simulink model "Simulatore_COMAU_UBHAND_IV_manipolazione_interna"  (Simulink 7.6)
settings: Solver=ode45  StartTime=0.0  StopTime=inf  RelTol=1e-3
values from neighbouring files (symbols this model uses):
  a_f = zeros(4,5)    [KinematicData.m]
  alpha_f = zeros(4,5)    [KinematicData.m]
  d_f = zeros(4,5)    [KinematicData.m]
  dc4_1 = 660    [KinematicData.m]
  T6HAND = [1 0 0 0;0 1 0 0; 0 0 1 357.48+95; 0 0 0 1]    [KinematicData.m]
  teta_d = atan(647.07/130)    [KinematicData.m]
  teta_f = zeros(4,5)    [KinematicData.m]
undefined symbols (not defined in the file or in its folder): a_c, a_w, alpha_c, alpha_w, d_c, d_w, Dt_fingers, teta_c
libraries: simulink x281, dspmtrx3 x171, aerolibutil x163, aerolibconvert2 x64, simulink_extras x46, vrlib x26, aerolibtransform2 x7, dspnetwork x1
(part 10 of 15)

== system Simulatore_COMAU_UBHAND_IV_manipolazione_interna/Work Space Control/Grasp Planner/box/punti di contatto box/punti di contatto sfera/Subsystem3 ==
  dimens1/2/side : Inport
  T_object : Inport  Port=2
  Constant : Constant
  Demux : Demux  Outputs=3
  Demux2 : Demux  Outputs=3
  Demux3 : Demux  Outputs=3
  Display : Display
  Display1 : Display
  Gain : Gain  Gain=-1
  Gain1 : Gain  Gain=-1
  Gain2 : Gain  Gain=-1
  Gain3 : Gain  Gain=1/2
  Matrix Multiply : Product  Multiplication=Matrix(*)
  Multiport Switch : MultiPortSwitch  Inputs=6  zeroidx=off
  Mux : Mux  Inputs=2
  Mux{1..6} (6 alike) : Mux  Inputs=3
  R_0EE1 : dspmtrx3/Submatrix  RowSpan=Range of rows  RowStartMode=Index  RowStartIndex=1  RowEndMode=Index  RowEndIndex=3  ColSpan=One column  ColStartMode=Index  ColStartIndex=4  ColEndMode=Index  ColEndIndex=4
  R_0EE2 : dspmtrx3/Submatrix  RowSpan=Range of rows  RowStartMode=Index  RowStartIndex=1  RowEndMode=Index  RowEndIndex=3  ColSpan=Range of columns  ColStartMode=Index  ColStartIndex=1  ColEndMode=Index  ColEndIndex=3
  Sum : Sum  Inputs=|++
  Sum3 : Sum
  Sum4 : Sum  Inputs=|++
  Sum5 : Sum  Inputs=|++
  dimens1/2/side1 : InportShadow  Port=1
  xyz 0 : Outport
  wires:
  R_0EE1/1 -> Sum3/2
  R_0EE2/1 -> Matrix Multiply/1
  T_object/1 -> R_0EE1/1, R_0EE2/1
  Multiport Switch/1 -> Matrix Multiply/2
  Matrix Multiply/1 -> Sum3/1
  [dim2] -> Mux5/2
  Gain1/1 -> Mux6/3
  [dim2] -> Mux6/2
  Gain3/1 -> Display1/1, Demux/1
  Demux/1 -> Sum5/1
  Demux/3 -> Sum/2
  [dim2] -> Mux3/3
  [dim2] -> Mux4/3
  Gain2/1 -> Mux4/2
  Mux4/1 -> Multiport Switch/7
  Mux3/1 -> Multiport Switch/6
  Mux6/1 -> Multiport Switch/5
  Mux5/1 -> Multiport Switch/4
  Mux2/1 -> Multiport Switch/3
  Mux1/1 -> Multiport Switch/2
  [dim1] -> Mux4/1
  [dim1] -> Mux3/1
  [dim1] -> Mux6/1
  [dim1] -> Mux5/1
  [dim1] -> Mux2/2
  [dim2] -> Mux2/3
  [dim1] -> Mux1/2
  [dim2] -> Mux1/3
  Demux3/1 -> [dim1]
  Demux3/2 -> [dim2]
  [radius_spheres] -> Sum5/2
  Sum5/1 -> Mux3/2, Gain2/1
  Sum4/1 -> Mux5/3, Gain1/1
  Demux/2 -> Sum4/2
  [radius_spheres] -> Sum4/1
  Gain/1 -> Mux2/1
  Sum/1 -> Display/1, Gain/1, Mux1/1
  dimens1/2/side1/1 -> Demux3/1
  Demux2/3 -> Multiport Switch/1
  Constant/1 -> Mux/2
  dimens1/2/side/1 -> Demux2/1
  Sum3/1 -> Mux/1
  Mux/1 -> xyz 0/1
  [radius_spheres] -> Sum/1
  [box_size] -> Gain3/1

== system Simulatore_COMAU_UBHAND_IV_manipolazione_interna/Work Space Control/Grasp Planner/box/punti di contatto box/punti di contatto sfera/Subsystem4 ==
  dimens1/2/side : Inport
  T_object : Inport  Port=2
  Constant : Constant
  Demux : Demux  Outputs=3
  Demux2 : Demux  Outputs=3
  Demux3 : Demux  Outputs=3
  Display : Display
  Display1 : Display
  Gain : Gain  Gain=-1
  Gain1 : Gain  Gain=-1
  Gain2 : Gain  Gain=-1
  Gain3 : Gain  Gain=1/2
  Matrix Multiply : Product  Multiplication=Matrix(*)
  Multiport Switch : MultiPortSwitch  Inputs=6  zeroidx=off
  Mux : Mux  Inputs=2
  Mux{1..6} (6 alike) : Mux  Inputs=3
  R_0EE1 : dspmtrx3/Submatrix  RowSpan=Range of rows  RowStartMode=Index  RowStartIndex=1  RowEndMode=Index  RowEndIndex=3  ColSpan=One column  ColStartMode=Index  ColStartIndex=4  ColEndMode=Index  ColEndIndex=4
  R_0EE2 : dspmtrx3/Submatrix  RowSpan=Range of rows  RowStartMode=Index  RowStartIndex=1  RowEndMode=Index  RowEndIndex=3  ColSpan=Range of columns  ColStartMode=Index  ColStartIndex=1  ColEndMode=Index  ColEndIndex=3
  Sum : Sum  Inputs=|++
  Sum3 : Sum
  Sum4 : Sum  Inputs=|++
  Sum5 : Sum  Inputs=|++
  dimens1/2/side1 : InportShadow  Port=1
  xyz 0 : Outport
  wires:
  [box_size] -> Gain3/1
  [radius_spheres] -> Sum/1
  Mux/1 -> xyz 0/1
  Sum3/1 -> Mux/1
  dimens1/2/side/1 -> Demux2/1
  Constant/1 -> Mux/2
  Demux2/3 -> Multiport Switch/1
  dimens1/2/side1/1 -> Demux3/1
  Sum/1 -> Mux1/1, Gain/1, Display/1
  Gain/1 -> Mux2/1
  [radius_spheres] -> Sum4/1
  Demux/2 -> Sum4/2
  Sum4/1 -> Gain1/1, Mux5/3
  Sum5/1 -> Gain2/1, Mux3/2
  [radius_spheres] -> Sum5/2
  Demux3/2 -> [dim2]
  Demux3/1 -> [dim1]
  [dim2] -> Mux1/3
  [dim1] -> Mux1/2
  [dim2] -> Mux2/3
  [dim1] -> Mux2/2
  [dim1] -> Mux5/1
  [dim1] -> Mux6/1
  [dim1] -> Mux3/1
  [dim1] -> Mux4/1
  Mux1/1 -> Multiport Switch/2
  Mux2/1 -> Multiport Switch/3
  Mux5/1 -> Multiport Switch/4
  Mux6/1 -> Multiport Switch/5
  Mux3/1 -> Multiport Switch/6
  Mux4/1 -> Multiport Switch/7
  Gain2/1 -> Mux4/2
  [dim2] -> Mux4/3
  [dim2] -> Mux3/3
  Demux/3 -> Sum/2
  Demux/1 -> Sum5/1
  Gain3/1 -> Demux/1, Display1/1
  [dim2] -> Mux6/2
  Gain1/1 -> Mux6/3
  [dim2] -> Mux5/2
  Matrix Multiply/1 -> Sum3/1
  Multiport Switch/1 -> Matrix Multiply/2
  T_object/1 -> R_0EE2/1, R_0EE1/1
  R_0EE2/1 -> Matrix Multiply/1
  R_0EE1/1 -> Sum3/2

== system Simulatore_COMAU_UBHAND_IV_manipolazione_interna/Work Space Control/Grasp Planner/cilindro/Subsystem/R_end_effector/rot_x ==
  alpha : Inport
  Angle Conversion : aerolibconvert2/Angle Conversion  IU=deg  OU=rad
  Constant{,1..2,5} (4 alike) : Constant  Value=0
  Constant4 : Constant
  Create 3x3 Matrix : aerolibutil/Create 3x3 Matrix
  Gain : Gain  Gain=-1
  Trigonometric Function : Trigonometry
  Trigonometric Function1 : Trigonometry  Operator=cos
  Trigonometric Function2 : Trigonometry
  Trigonometric Function3 : Trigonometry  Operator=cos
  rotx : Outport
  wires:
  alpha/1 -> Angle Conversion/1
  Angle Conversion/1 -> Trigonometric Function3/1, Trigonometric Function2/1, Trigonometric Function/1, Trigonometric Function1/1
  Create 3x3 Matrix/1 -> rotx/1
  Constant4/1 -> Create 3x3 Matrix/1
  Constant2/1 -> Create 3x3 Matrix/2
  Constant/1 -> Create 3x3 Matrix/3
  Constant5/1 -> Create 3x3 Matrix/4
  Trigonometric Function3/1 -> Create 3x3 Matrix/5
  Trigonometric Function2/1 -> Gain/1
  Gain/1 -> Create 3x3 Matrix/6
  Constant1/1 -> Create 3x3 Matrix/7
  Trigonometric Function/1 -> Create 3x3 Matrix/8
  Trigonometric Function1/1 -> Create 3x3 Matrix/9

== system Simulatore_COMAU_UBHAND_IV_manipolazione_interna/Work Space Control/Grasp Planner/cilindro/Subsystem/R_end_effector/rot_y ==
  beta : Inport
  Angle Conversion : aerolibconvert2/Angle Conversion  IU=deg  OU=rad
  Constant{1..3,5} (4 alike) : Constant  Value=0
  Constant4 : Constant
  Create 3x3 Matrix : aerolibutil/Create 3x3 Matrix
  Gain : Gain  Gain=-1
  Trigonometric Function : Trigonometry
  Trigonometric Function1 : Trigonometry  Operator=cos
  Trigonometric Function4 : Trigonometry  Operator=cos
  Trigonometric Function5 : Trigonometry
  roty : Outport
  wires:
  Constant1/1 -> Create 3x3 Matrix/8
  Gain/1 -> Create 3x3 Matrix/7
  Trigonometric Function4/1 -> Create 3x3 Matrix/1
  Trigonometric Function1/1 -> Create 3x3 Matrix/9
  Trigonometric Function/1 -> Gain/1
  Constant3/1 -> Create 3x3 Matrix/6
  Constant4/1 -> Create 3x3 Matrix/5
  Constant5/1 -> Create 3x3 Matrix/4
  Trigonometric Function5/1 -> Create 3x3 Matrix/3
  Constant2/1 -> Create 3x3 Matrix/2
  Create 3x3 Matrix/1 -> roty/1
  Angle Conversion/1 -> Trigonometric Function4/1, Trigonometric Function5/1, Trigonometric Function/1, Trigonometric Function1/1
  beta/1 -> Angle Conversion/1

== system Simulatore_COMAU_UBHAND_IV_manipolazione_interna/Work Space Control/Grasp Planner/cilindro/Subsystem/R_end_effector/rot_z ==
  gamma : Inport
  Angle Conversion : aerolibconvert2/Angle Conversion  IU=deg  OU=rad
  Constant{,1..3} (4 alike) : Constant  Value=0
  Constant4 : Constant
  Create 3x3 Matrix : aerolibutil/Create 3x3 Matrix
  Gain1 : Gain  Gain=-1
  Trigonometric Function3 : Trigonometry  Operator=cos
  Trigonometric Function4 : Trigonometry  Operator=cos
  Trigonometric Function5 : Trigonometry
  Trigonometric Function6 : Trigonometry
  rotz : Outport
  wires:
  Trigonometric Function5/1 -> Gain1/1
  Constant4/1 -> Create 3x3 Matrix/9
  Constant3/1 -> Create 3x3 Matrix/8
  Constant1/1 -> Create 3x3 Matrix/7
  Constant2/1 -> Create 3x3 Matrix/6
  Trigonometric Function3/1 -> Create 3x3 Matrix/5
  Trigonometric Function6/1 -> Create 3x3 Matrix/4
  Constant/1 -> Create 3x3 Matrix/3
  Gain1/1 -> Create 3x3 Matrix/2
  Trigonometric Function4/1 -> Create 3x3 Matrix/1
  Create 3x3 Matrix/1 -> rotz/1
  Angle Conversion/1 -> Trigonometric Function4/1, Trigonometric Function5/1, Trigonometric Function6/1, Trigonometric Function3/1
  gamma/1 -> Angle Conversion/1

== system Simulatore_COMAU_UBHAND_IV_manipolazione_interna/Work Space Control/Grasp Planner/cilindro/punti di contatto cilindro/object rotation matrix/R ==
  alpha : Inport
  beta : Inport  Port=2
  gamma : Inport  Port=3
  Matrix Multiply3 : Product  Multiplication=Matrix(*)
  Matrix Multiply4 : Product  Multiplication=Matrix(*)
  rot_x : Subsystem
  rot_y : Subsystem
  rot_z : Subsystem
  R : Outport
  wires:
  gamma/1 -> rot_z/1(gamma)
  beta/1 -> rot_y/1(beta)
  alpha/1 -> rot_x/1(alpha)
  rot_x/1(rotx) -> Matrix Multiply3/1
  rot_y/1(roty) -> Matrix Multiply3/2
  rot_z/1(rotz) -> Matrix Multiply4/2
  Matrix Multiply3/1 -> Matrix Multiply4/1
  Matrix Multiply4/1 -> R/1

== system Simulatore_COMAU_UBHAND_IV_manipolazione_interna/Work Space Control/Grasp Planner/cilindro/punti di contatto cilindro/object rotation matrix/R_01 ==
  alpha : Inport
  beta : Inport  Port=2
  gamma : Inport  Port=3
  Matrix Multiply3 : Product  Multiplication=Matrix(*)
  Matrix Multiply4 : Product  Multiplication=Matrix(*)
  rot_x : Subsystem
  rot_y : Subsystem
  rot_z : Subsystem
  R_01 : Outport
  wires:
  gamma/1 -> rot_z/1(gamma)
  beta/1 -> rot_y/1(beta)
  alpha/1 -> rot_x/1(alpha)
  rot_x/1(rotx) -> Matrix Multiply3/1
  rot_y/1(roty) -> Matrix Multiply3/2
  rot_z/1(rotz) -> Matrix Multiply4/2
  Matrix Multiply3/1 -> Matrix Multiply4/1
  Matrix Multiply4/1 -> R_01/1

== system Simulatore_COMAU_UBHAND_IV_manipolazione_interna/Work Space Control/Grasp Planner/cilindro/punti di contatto cilindro/object rotation matrix/R_56 ==
  alpha : Inport
  beta : Inport  Port=2
  gamma : Inport  Port=3
  Matrix Multiply3 : Product  Multiplication=Matrix(*)
  Matrix Multiply4 : Product  Multiplication=Matrix(*)
  rot_x : Subsystem
  rot_y : Subsystem
  rot_z : Subsystem
  R56 : Outport
  wires:
  Matrix Multiply4/1 -> R56/1
  Matrix Multiply3/1 -> Matrix Multiply4/1
  rot_z/1(rotz) -> Matrix Multiply4/2
  rot_y/1(roty) -> Matrix Multiply3/2
  rot_x/1(rotx) -> Matrix Multiply3/1
  alpha/1 -> rot_x/1(alpha)
  beta/1 -> rot_y/1(beta)
  gamma/1 -> rot_z/1(gamma)

== system Simulatore_COMAU_UBHAND_IV_manipolazione_interna/Work Space Control/Grasp Planner/cilindro/punti di contatto cilindro/object rotation matrix/Subsystem ==
  R : Inport
  p : Inport  Port=2
  Constant1 : Constant  Value=[0 0 0 1]  VectorParams1D=off
  Matrix Concatenate : Concatenate  Mode=Multidimensional array  ConcatenateDimension=2
  Matrix Concatenate1 : Concatenate  Mode=Multidimensional array
  T : Outport
  wires:
  Constant1/1 -> Matrix Concatenate1/2
  Matrix Concatenate/1 -> Matrix Concatenate1/1
  p/1 -> Matrix Concatenate/2
  R/1 -> Matrix Concatenate/1
  Matrix Concatenate1/1 -> T/1

== system Simulatore_COMAU_UBHAND_IV_manipolazione_interna/Work Space Control/Grasp Planner/cilindro/punti di contatto cilindro/object rotation matrix/Subsystem1 ==
  R : Inport
  p : Inport  Port=2
  Constant2 : Constant  Value=[0 0 0 1]  VectorParams1D=off
  Matrix Concatenate2 : Concatenate  Mode=Multidimensional array  ConcatenateDimension=2
  Matrix Concatenate3 : Concatenate  Mode=Multidimensional array
  T : Outport
  wires:
  Constant2/1 -> Matrix Concatenate3/2
  Matrix Concatenate2/1 -> Matrix Concatenate3/1
  R/1 -> Matrix Concatenate2/1
  p/1 -> Matrix Concatenate2/2
  Matrix Concatenate3/1 -> T/1

== system Simulatore_COMAU_UBHAND_IV_manipolazione_interna/Work Space Control/Grasp Planner/cilindro/punti di contatto cilindro/object rotation matrix/Subsystem2 ==
  R : Inport
  p : Inport  Port=2
  Constant1 : Constant  Value=[0 0 0 1]  VectorParams1D=off
  Matrix Concatenate : Concatenate  Mode=Multidimensional array  ConcatenateDimension=2
  Matrix Concatenate1 : Concatenate  Mode=Multidimensional array
  T : Outport
  wires:
  Matrix Concatenate1/1 -> T/1
  R/1 -> Matrix Concatenate/1
  p/1 -> Matrix Concatenate/2
  Matrix Concatenate/1 -> Matrix Concatenate1/1
  Constant1/1 -> Matrix Concatenate1/2

== system Simulatore_COMAU_UBHAND_IV_manipolazione_interna/Work Space Control/Grasp Planner/cilindro/punti di contatto cilindro/object rotation matrix/definizione_T_0_object_tip1 ==
  cilindro_pos : Inport
  R_01 : Inport  Port=2
  1pc : Inport  Port=3
  2pr : Inport  Port=4
  3pc : Inport  Port=5
  R : Inport  Port=6
  1pc1 : InportShadow  Port=3
  Display : Display
  Display1 : Display
  Matrix Multiply{1..6,8..9} (8 alike) : Product  Multiplication=Matrix(*)
  Multiport Switch1 : MultiPortSwitch  Inputs=2  zeroidx=off
  R_1 : Constant  Value=eye(3)
  R_12 : Constant  Value=eye(3)
  Sum1 : Sum
  Sum3 : Sum  Inputs=+-
  Sum4 : Sum
  Sum5 : Sum
  0pc : Outport
  0pr : Outport  Port=2
  0pc' : Outport  Port=3
  R1 : Outport  Port=4
  R_05 : Outport  Port=5
  tag scope: reference_point_cilindro=global
  wires:
  R_01/1 -> Matrix Multiply9/2, Matrix Multiply1/1, Matrix Multiply2/1, Matrix Multiply3/1, Multiport Switch1/2
  1pc/1 -> Matrix Multiply1/2
  cilindro_pos/1 -> Sum3/1, Display/1
  Matrix Multiply1/1 -> Sum3/2, Display1/1
  Sum3/1 -> 0pc/1, Sum4/1
  R_12/1 -> Matrix Multiply2/2
  Matrix Multiply2/1 -> Matrix Multiply5/1
  2pr/1 -> Matrix Multiply3/2
  Matrix Multiply3/1 -> Sum4/2
  Sum4/1 -> 0pr/1, Sum5/1
  R_1/1 -> Matrix Multiply5/2
  Sum5/1 -> 0pc'/1, Sum1/1
  Matrix Multiply8/1 -> Sum5/2
  Matrix Multiply9/1 -> R_05/1, Multiport Switch1/3
  R/1 -> Matrix Multiply8/1, Matrix Multiply9/1, R1/1
  3pc/1 -> Matrix Multiply6/2
  Matrix Multiply6/1 -> Matrix Multiply8/2
  Sum1/1 -> [reference_point_cilindro]
  Matrix Multiply4/1 -> Sum1/2
  1pc1/1 -> Matrix Multiply4/2
  Matrix Multiply5/1 -> Matrix Multiply6/1
  [orientation_mode_cilindro] -> Multiport Switch1/1
  Multiport Switch1/1 -> Matrix Multiply4/1

== system Simulatore_COMAU_UBHAND_IV_manipolazione_interna/Work Space Control/Grasp Planner/cilindro/punti di contatto cilindro/object rotation matrix/definizione_T_0_object_tip2 ==
  cilindro_pos : Inport
  R_01 : Inport  Port=2
  1pc : Inport  Port=3
  2pr : Inport  Port=4
  3pc : Inport  Port=5
  R : Inport  Port=6
  1pc1 : InportShadow  Port=3
  Matrix Multiply{1..6,8..9} (8 alike) : Product  Multiplication=Matrix(*)
  Multiport Switch1 : MultiPortSwitch  Inputs=2  zeroidx=off
  R_1 : Constant  Value=eye(3)
  R_12 : Constant  Value=eye(3)
  Sum1 : Sum
  Sum3 : Sum  Inputs=+-
  Sum4 : Sum
  Sum5 : Sum
  0pc : Outport
  0pr : Outport  Port=2
  0pc' : Outport  Port=3
  R1 : Outport  Port=4
  R_05 : Outport  Port=5
  wires:
  Multiport Switch1/1 -> Matrix Multiply4/1
  [orientation_mode_cilindro] -> Multiport Switch1/1
  Matrix Multiply5/1 -> Matrix Multiply6/1
  1pc1/1 -> Matrix Multiply4/2
  Matrix Multiply4/1 -> Sum1/2
  Matrix Multiply6/1 -> Matrix Multiply8/2
  3pc/1 -> Matrix Multiply6/2
  R/1 -> R1/1, Matrix Multiply9/1, Matrix Multiply8/1
  Matrix Multiply9/1 -> Multiport Switch1/3, R_05/1
  Matrix Multiply8/1 -> Sum5/2
  Sum5/1 -> Sum1/1, 0pc'/1
  R_1/1 -> Matrix Multiply5/2
  Sum4/1 -> Sum5/1, 0pr/1
  Matrix Multiply3/1 -> Sum4/2
  2pr/1 -> Matrix Multiply3/2
  Matrix Multiply2/1 -> Matrix Multiply5/1
  R_12/1 -> Matrix Multiply2/2
  Sum3/1 -> Sum4/1, 0pc/1
  Matrix Multiply1/1 -> Sum3/2
  cilindro_pos/1 -> Sum3/1
  1pc/1 -> Matrix Multiply1/2
  R_01/1 -> Multiport Switch1/2, Matrix Multiply3/1, Matrix Multiply2/1, Matrix Multiply1/1, Matrix Multiply9/2

== system Simulatore_COMAU_UBHAND_IV_manipolazione_interna/Work Space Control/Grasp Planner/cilindro/punti di contatto cilindro/punti di contatto cilindro/Subsystem1 ==
  teta/z : Inport
  T_object : Inport  Port=2
  offset : Inport  Port=3
  object_manipulation : Inport  Port=4
  Constant : Constant
  Constant1 : Constant
  Constant2 : Constant  Value=eye(3,3)  VectorParams1D=off
  Constant4 : Constant
  Demux : Demux  Outputs=2
  Demux2 : Demux  Outputs=2
  Display{,1..3} (4 alike) : Display
  Gain : Gain  Gain=1/2
  Matrix Multiply{1..5} (5 alike) : Product  Multiplication=Matrix(*)
  Mux1 : Mux  Inputs=2
  Mux2 : Mux  Inputs=2
  Mux3 : Mux  Inputs=3
  Polar to Cartesian : simulink_extras/Transformations/Polar to Cartesian
  Subsystem1 : Subsystem
  Sum : Sum  Inputs=|+++
  T^-1 : Subsystem
  index_xyz_0 : Outport
  xyz 0 : Outport  Port=2
  wires:
  T_object/1 -> Matrix Multiply1/1, Matrix Multiply4/1
  Polar to Cartesian/1 -> Mux3/1
  Polar to Cartesian/2 -> Mux3/2
  Demux2/2 -> Mux3/3
  teta/z/1 -> Demux2/1
  Demux2/1 -> Polar to Cartesian/2
  [radius_spheres] -> Gain/1
  Demux/2 -> Sum/2
  Sum/1 -> Polar to Cartesian/1
  [cilindro_size] -> Demux/1
  Constant1/1 -> Mux2/2
  Gain/1 -> Sum/1
  offset/1 -> Sum/3
  Constant/1 -> [one]
  Mux3/1 -> Mux1/1, Mux2/1
  Matrix Multiply3/1 -> Display1/1, xyz 0/1
  Constant2/1 -> Subsystem1/1(R)
  [reference_point] -> Subsystem1/2(p)
  Subsystem1/1(T) -> T^-1/1(T), Matrix Multiply4/2
  T^-1/1(T^-1) -> Matrix Multiply2/1
  object_manipulation/1 -> Matrix Multiply5/1
  Matrix Multiply2/1 -> Matrix Multiply5/2, Display2/1
  Constant4/1 -> Mux1/2
  Mux1/1 -> Matrix Multiply2/2
  Matrix Multiply5/1 -> Matrix Multiply3/2, Display3/1
  Matrix Multiply4/1 -> Matrix Multiply3/1
  Mux2/1 -> Matrix Multiply1/2
  Matrix Multiply1/1 -> index_xyz_0/1

== system Simulatore_COMAU_UBHAND_IV_manipolazione_interna/Work Space Control/Grasp Planner/cilindro/punti di contatto cilindro/punti di contatto cilindro/Subsystem2 ==
  teta/z : Inport
  T_object : Inport  Port=2
  offset : Inport  Port=3
  object_manipulation : Inport  Port=4
  Constant2 : Constant
  Constant3 : Constant  Value=eye(3,3)  VectorParams1D=off
  Demux : Demux  Outputs=2
  Demux2 : Demux  Outputs=2
  Display{1..4} (4 alike) : Display
  Gain : Gain  Gain=1/2
  Matrix Multiply{1..4} (4 alike) : Product  Multiplication=Matrix(*)
  Mux3 : Mux
  Polar to Cartesian : simulink_extras/Transformations/Polar to Cartesian
  Subsystem1 : Subsystem
  Sum : Sum  Inputs=|+++
  T^-1 : Subsystem
  xyz 0 : Outport
  wires:
  [cilindro_size] -> Demux/1
  Sum/1 -> Polar to Cartesian/1
  Demux/2 -> Sum/2
  [radius_spheres] -> Gain/1
  Demux2/1 -> Polar to Cartesian/2
  teta/z/1 -> Demux2/1
  Demux2/2 -> Mux3/3
  Polar to Cartesian/2 -> Mux3/2
  Polar to Cartesian/1 -> Mux3/1
  T_object/1 -> Matrix Multiply1/1
  Gain/1 -> Sum/1
  offset/1 -> Sum/3
  Constant2/1 -> Mux3/4
  Constant3/1 -> Subsystem1/1(R)
  [reference_point] -> Subsystem1/2(p)
  Subsystem1/1(T) -> T^-1/1(T), Matrix Multiply1/2
  T^-1/1(T^-1) -> Matrix Multiply2/1
  Mux3/1 -> Matrix Multiply2/2, Display1/1
  Matrix Multiply2/1 -> Matrix Multiply4/2, Display2/1
  object_manipulation/1 -> Matrix Multiply4/1
  Matrix Multiply4/1 -> Display3/1, Matrix Multiply3/2
  Matrix Multiply1/1 -> Matrix Multiply3/1
  Matrix Multiply3/1 -> Display4/1, xyz 0/1

== system Simulatore_COMAU_UBHAND_IV_manipolazione_interna/Work Space Control/Grasp Planner/cilindro/punti di contatto cilindro/punti di contatto cilindro/Subsystem3 ==
  teta/z : Inport
  T_object : Inport  Port=2
  offset : Inport  Port=3
  object_manipulation : Inport  Port=4
  Constant2 : Constant
  Constant3 : Constant  Value=eye(3,3)  VectorParams1D=off
  Demux : Demux  Outputs=2
  Demux2 : Demux  Outputs=2
  Display1 : Display
  Gain : Gain  Gain=1/2
  Matrix Multiply1 : Product  Inputs=3  Multiplication=Matrix(*)
  Matrix Multiply2 : Product  Multiplication=Matrix(*)
  Matrix Multiply4 : Product  Multiplication=Matrix(*)
  Mux3 : Mux
  Polar to Cartesian : simulink_extras/Transformations/Polar to Cartesian
  Subsystem1 : Subsystem
  Sum : Sum  Inputs=|+++
  T^-1 : Subsystem
  xyz 0 : Outport
  wires:
  Matrix Multiply4/1 -> Matrix Multiply1/3
  object_manipulation/1 -> Matrix Multiply4/1
  Matrix Multiply2/1 -> Matrix Multiply4/2
  Mux3/1 -> Matrix Multiply2/2
  T^-1/1(T^-1) -> Matrix Multiply2/1
  Subsystem1/1(T) -> Matrix Multiply1/2, T^-1/1(T)
  [reference_point] -> Subsystem1/2(p)
  Constant3/1 -> Subsystem1/1(R)
  Constant2/1 -> Mux3/4
  offset/1 -> Sum/3
  Gain/1 -> Sum/1
  Matrix Multiply1/1 -> xyz 0/1
  T_object/1 -> Matrix Multiply1/1
  Polar to Cartesian/1 -> Mux3/1
  Polar to Cartesian/2 -> Mux3/2
  Demux2/2 -> Mux3/3
  teta/z/1 -> Demux2/1
  Demux2/1 -> Polar to Cartesian/2
  [radius_spheres] -> Gain/1
  Demux/2 -> Sum/2
  Sum/1 -> Polar to Cartesian/1
  [cilindro_size] -> Demux/1

== system Simulatore_COMAU_UBHAND_IV_manipolazione_interna/Work Space Control/Grasp Planner/cilindro/punti di contatto cilindro/punti di contatto cilindro/Subsystem4 ==
  teta/z : Inport
  T_object : Inport  Port=2
  offset : Inport  Port=3
  object_manipulation : Inport  Port=4
  Constant2 : Constant
  Constant3 : Constant  Value=eye(3,3)  VectorParams1D=off
  Demux : Demux  Outputs=2
  Demux2 : Demux  Outputs=2
  Display1 : Display
  Gain : Gain  Gain=1/2
  Matrix Multiply1 : Product  Inputs=3  Multiplication=Matrix(*)
  Matrix Multiply2 : Product  Multiplication=Matrix(*)
  Matrix Multiply4 : Product  Multiplication=Matrix(*)
  Mux3 : Mux
  Polar to Cartesian : simulink_extras/Transformations/Polar to Cartesian
  Subsystem1 : Subsystem
  Sum : Sum  Inputs=|+++
  T^-1 : Subsystem
  xyz 0 : Outport
  wires:
  [cilindro_size] -> Demux/1
  Sum/1 -> Polar to Cartesian/1
  Demux/2 -> Sum/2
  [radius_spheres] -> Gain/1
  Demux2/1 -> Polar to Cartesian/2
  teta/z/1 -> Demux2/1
  Demux2/2 -> Mux3/3
  Polar to Cartesian/2 -> Mux3/2
  Polar to Cartesian/1 -> Mux3/1
  T_object/1 -> Matrix Multiply1/1
  Matrix Multiply1/1 -> xyz 0/1
  Gain/1 -> Sum/1
  offset/1 -> Sum/3
  Constant2/1 -> Mux3/4
  Constant3/1 -> Subsystem1/1(R)
  [reference_point] -> Subsystem1/2(p)
  Subsystem1/1(T) -> T^-1/1(T), Matrix Multiply1/2
  T^-1/1(T^-1) -> Matrix Multiply2/1
  Mux3/1 -> Matrix Multiply2/2
  Matrix Multiply2/1 -> Matrix Multiply4/2
  object_manipulation/1 -> Matrix Multiply4/1
  Matrix Multiply4/1 -> Matrix Multiply1/3

== system Simulatore_COMAU_UBHAND_IV_manipolazione_interna/Work Space Control/Grasp Planner/cilindro/punti di contatto cilindro/punti di contatto cilindro/Subsystem5 ==
  teta/z : Inport
  T_object : Inport  Port=2
  offset : Inport  Port=3
  object_manipulation : Inport  Port=4
  Constant2 : Constant
  Constant3 : Constant  Value=eye(3,3)  VectorParams1D=off
  Demux : Demux  Outputs=2
  Demux2 : Demux  Outputs=2
  Display1 : Display
  Gain : Gain  Gain=1/2
  Matrix Multiply1 : Product  Inputs=3  Multiplication=Matrix(*)
  Matrix Multiply2 : Product  Multiplication=Matrix(*)
  Matrix Multiply4 : Product  Multiplication=Matrix(*)
  Mux3 : Mux
  Polar to Cartesian : simulink_extras/Transformations/Polar to Cartesian
  Subsystem1 : Subsystem
  Sum : Sum  Inputs=|+++
  T^-1 : Subsystem
  xyz 0 : Outport
  wires:
  Matrix Multiply4/1 -> Matrix Multiply1/3
  object_manipulation/1 -> Matrix Multiply4/1
  Matrix Multiply2/1 -> Matrix Multiply4/2
  Mux3/1 -> Matrix Multiply2/2
  T^-1/1(T^-1) -> Matrix Multiply2/1
  Subsystem1/1(T) -> Matrix Multiply1/2, T^-1/1(T)
  [reference_point] -> Subsystem1/2(p)
  Constant3/1 -> Subsystem1/1(R)
  Constant2/1 -> Mux3/4
  offset/1 -> Sum/3
  Gain/1 -> Sum/1
  Matrix Multiply1/1 -> xyz 0/1
  T_object/1 -> Matrix Multiply1/1
  Polar to Cartesian/1 -> Mux3/1
  Polar to Cartesian/2 -> Mux3/2
  Demux2/2 -> Mux3/3
  teta/z/1 -> Demux2/1
  Demux2/1 -> Polar to Cartesian/2
  [radius_spheres] -> Gain/1
  Demux/2 -> Sum/2
  Sum/1 -> Polar to Cartesian/1
  [cilindro_size] -> Demux/1

== system Simulatore_COMAU_UBHAND_IV_manipolazione_interna/Work Space Control/Grasp Planner/sfera/punti di contatto sfera/object rotation matrix/R ==
  alpha : Inport
  beta : Inport  Port=2
  gamma : Inport  Port=3
  Matrix Multiply3 : Product  Multiplication=Matrix(*)
  Matrix Multiply4 : Product  Multiplication=Matrix(*)
  rot_x : Subsystem
  rot_y : Subsystem
  rot_z : Subsystem
  R : Outport
  wires:
  gamma/1 -> rot_z/1(gamma)
  beta/1 -> rot_y/1(beta)
  alpha/1 -> rot_x/1(alpha)
  rot_x/1(rotx) -> Matrix Multiply3/1
  rot_y/1(roty) -> Matrix Multiply3/2
  rot_z/1(rotz) -> Matrix Multiply4/2
  Matrix Multiply3/1 -> Matrix Multiply4/1
  Matrix Multiply4/1 -> R/1

== system Simulatore_COMAU_UBHAND_IV_manipolazione_interna/Work Space Control/Grasp Planner/sfera/punti di contatto sfera/object rotation matrix/R_01 ==
  alpha : Inport
  beta : Inport  Port=2
  gamma : Inport  Port=3
  Matrix Multiply3 : Product  Multiplication=Matrix(*)
  Matrix Multiply4 : Product  Multiplication=Matrix(*)
  rot_x : Subsystem
  rot_y : Subsystem
  rot_z : Subsystem
  R_01 : Outport
  wires:
  gamma/1 -> rot_z/1(gamma)
  beta/1 -> rot_y/1(beta)
  alpha/1 -> rot_x/1(alpha)
  rot_x/1(rotx) -> Matrix Multiply3/1
  rot_y/1(roty) -> Matrix Multiply3/2
  rot_z/1(rotz) -> Matrix Multiply4/2
  Matrix Multiply3/1 -> Matrix Multiply4/1
  Matrix Multiply4/1 -> R_01/1

== system Simulatore_COMAU_UBHAND_IV_manipolazione_interna/Work Space Control/Grasp Planner/sfera/punti di contatto sfera/object rotation matrix/R_56 ==
  alpha : Inport
  beta : Inport  Port=2
  gamma : Inport  Port=3
  Matrix Multiply3 : Product  Multiplication=Matrix(*)
  Matrix Multiply4 : Product  Multiplication=Matrix(*)
  rot_x : Subsystem
  rot_y : Subsystem
  rot_z : Subsystem
  R56 : Outport
  wires:
  Matrix Multiply4/1 -> R56/1
  Matrix Multiply3/1 -> Matrix Multiply4/1
  rot_z/1(rotz) -> Matrix Multiply4/2
  rot_y/1(roty) -> Matrix Multiply3/2
  rot_x/1(rotx) -> Matrix Multiply3/1
  alpha/1 -> rot_x/1(alpha)
  beta/1 -> rot_y/1(beta)
  gamma/1 -> rot_z/1(gamma)

== system Simulatore_COMAU_UBHAND_IV_manipolazione_interna/Work Space Control/Grasp Planner/sfera/punti di contatto sfera/object rotation matrix/definizione_T_0_object_tip1 ==
  sfera_pos : Inport
  R_01 : Inport  Port=2
  1pc : Inport  Port=3
  2pr : Inport  Port=4
  3pc : Inport  Port=5
  R : Inport  Port=6
  1pc1 : InportShadow  Port=3
  Display{,1..4} (5 alike) : Display
  Matrix Multiply{1..6,8..9} (8 alike) : Product  Multiplication=Matrix(*)
  Multiport Switch1 : MultiPortSwitch  Inputs=2  zeroidx=off
  R_1 : Constant  Value=eye(3)
  R_12 : Constant  Value=eye(3)
  Sum1 : Sum
  Sum3 : Sum  Inputs=+-
  Sum4 : Sum
  Sum5 : Sum
  0pc : Outport
  0pr : Outport  Port=2
  0pc' : Outport  Port=3
  R1 : Outport  Port=4
  R_05 : Outport  Port=5
  tag scope: reference_point_sfera=global
  wires:
  R_01/1 -> Matrix Multiply9/2, Matrix Multiply1/1, Matrix Multiply2/1, Matrix Multiply3/1, Multiport Switch1/2
  1pc/1 -> Matrix Multiply1/2
  sfera_pos/1 -> Sum3/1
  Matrix Multiply1/1 -> Sum3/2
  Sum3/1 -> 0pc/1, Sum4/1
  R_12/1 -> Matrix Multiply2/2
  Matrix Multiply2/1 -> Matrix Multiply5/1
  2pr/1 -> Matrix Multiply3/2
  Matrix Multiply3/1 -> Sum4/2, Display3/1
  Sum4/1 -> 0pr/1, Sum5/1, Display2/1
  R_1/1 -> Matrix Multiply5/2
  Sum5/1 -> Display/1, 0pc'/1, Sum1/1
  Matrix Multiply8/1 -> Display1/1, Sum5/2
  Matrix Multiply9/1 -> R_05/1, Multiport Switch1/3
  R/1 -> Matrix Multiply8/1, Matrix Multiply9/1, R1/1
  3pc/1 -> Matrix Multiply6/2
  Matrix Multiply6/1 -> Matrix Multiply8/2, Display4/1
  Sum1/1 -> [reference_point_sfera]
  Matrix Multiply4/1 -> Sum1/2
  1pc1/1 -> Matrix Multiply4/2
  Matrix Multiply5/1 -> Matrix Multiply6/1
  [orientation_mode_sfera] -> Multiport Switch1/1
  Multiport Switch1/1 -> Matrix Multiply4/1

== system Simulatore_COMAU_UBHAND_IV_manipolazione_interna/Work Space Control/Grasp Planner/sfera/punti di contatto sfera/punti di contatto sfera/R ==
  alpha,beta,gamma : Inport
  Constant1 : Constant  Value=[0 0 0 1]  VectorParams1D=off
  Constant2 : Constant  Value=[0 0 0]'  VectorParams1D=off
  Demux : Demux  Outputs=3
  Matrix Concatenate : Concatenate  Mode=Multidimensional array  ConcatenateDimension=2
  Matrix Concatenate1 : Concatenate  Mode=Multidimensional array
  Matrix Multiply3 : Product  Multiplication=Matrix(*)
  Matrix Multiply4 : Product  Multiplication=Matrix(*)
  rot_x : Subsystem
  rot_y : Subsystem
  rot_z : Subsystem
  T : Outport
  wires:
  Matrix Multiply3/1 -> Matrix Multiply4/1
  rot_z/1(rotz) -> Matrix Multiply4/2
  rot_y/1(roty) -> Matrix Multiply3/2
  rot_x/1(rotx) -> Matrix Multiply3/1
  alpha,beta,gamma/1 -> Demux/1
  Demux/3 -> rot_z/1(gamma)
  Demux/2 -> rot_y/1(beta)
  Demux/1 -> rot_x/1(alpha)
  Constant1/1 -> Matrix Concatenate1/2
  Matrix Concatenate/1 -> Matrix Concatenate1/1
  Matrix Concatenate1/1 -> T/1
  Constant2/1 -> Matrix Concatenate/2
  Matrix Multiply4/1 -> Matrix Concatenate/1

== system Simulatore_COMAU_UBHAND_IV_manipolazione_interna/Work Space Control/Grasp Planner/sfera/punti di contatto sfera/punti di contatto sfera/Subsystem ==
  teta/phi : Inport
  T_object : Inport  Port=2
  Constant : Constant
  Demux2 : Demux  Outputs=2
  Matrix Multiply1 : Product  Multiplication=Matrix(*)
  Mux : Mux  Inputs=2
  Mux1 : Mux  Inputs=3
  R_0EE1 : dspmtrx3/Submatrix  RowSpan=Range of rows  RowStartMode=Index  RowStartIndex=1  RowEndMode=Index  RowEndIndex=3  ColSpan=One column  ColStartMode=Index  ColStartIndex=4  ColEndMode=Index  ColEndIndex=4
  R_0EE2 : dspmtrx3/Submatrix  RowSpan=Range of rows  RowStartMode=Index  RowStartIndex=1  RowEndMode=Index  RowEndIndex=3  ColSpan=Range of columns  ColStartMode=Index  ColStartIndex=1  ColEndMode=Index  ColEndIndex=3
  Spherical to Cartesian : simulink_extras/Transformations/Spherical to Cartesian
  Sum : Sum  Inputs=|++
  Sum3 : Sum
  xyz 0 : Outport
  wires:
  [sfera_size] -> Sum/2
  [radius_spheres] -> Sum/1
  Mux/1 -> xyz 0/1
  Sum3/1 -> Mux/1
  teta/phi/1 -> Demux2/1
  Constant/1 -> Mux/2
  Demux2/2 -> Spherical to Cartesian/3
  Demux2/1 -> Spherical to Cartesian/2
  Sum/1 -> Spherical to Cartesian/1
  Spherical to Cartesian/1 -> Mux1/1
  Spherical to Cartesian/2 -> Mux1/2
  Spherical to Cartesian/3 -> Mux1/3
  Mux1/1 -> Matrix Multiply1/2
  R_0EE2/1 -> Matrix Multiply1/1
  T_object/1 -> R_0EE2/1, R_0EE1/1
  R_0EE1/1 -> Sum3/2
  Matrix Multiply1/1 -> Sum3/1

== system Simulatore_COMAU_UBHAND_IV_manipolazione_interna/Work Space Control/Grasp Planner/sfera/punti di contatto sfera/punti di contatto sfera/Subsystem1 ==
  teta/phi : Inport
  T_object : Inport  Port=2
  Constant : Constant
  Demux2 : Demux  Outputs=2
  Matrix Multiply1 : Product  Multiplication=Matrix(*)
  Mux : Mux  Inputs=2
  Mux1 : Mux  Inputs=3
  R_0EE1 : dspmtrx3/Submatrix  RowSpan=Range of rows  RowStartMode=Index  RowStartIndex=1  RowEndMode=Index  RowEndIndex=3  ColSpan=One column  ColStartMode=Index  ColStartIndex=4  ColEndMode=Index  ColEndIndex=4
  R_0EE2 : dspmtrx3/Submatrix  RowSpan=Range of rows  RowStartMode=Index  RowStartIndex=1  RowEndMode=Index  RowEndIndex=3  ColSpan=Range of columns  ColStartMode=Index  ColStartIndex=1  ColEndMode=Index  ColEndIndex=3
  Spherical to Cartesian : simulink_extras/Transformations/Spherical to Cartesian
  Sum : Sum  Inputs=|++
  Sum3 : Sum
  xyz 0 : Outport
  wires:
  Matrix Multiply1/1 -> Sum3/1
  R_0EE1/1 -> Sum3/2
  T_object/1 -> R_0EE1/1, R_0EE2/1
  R_0EE2/1 -> Matrix Multiply1/1
  Mux1/1 -> Matrix Multiply1/2
  Spherical to Cartesian/3 -> Mux1/3
  Spherical to Cartesian/2 -> Mux1/2
  Spherical to Cartesian/1 -> Mux1/1
  Sum/1 -> Spherical to Cartesian/1
  Demux2/1 -> Spherical to Cartesian/2
  Demux2/2 -> Spherical to Cartesian/3
  Constant/1 -> Mux/2
  teta/phi/1 -> Demux2/1
  Sum3/1 -> Mux/1
  Mux/1 -> xyz 0/1
  [radius_spheres] -> Sum/1
  [sfera_size] -> Sum/2

== system Simulatore_COMAU_UBHAND_IV_manipolazione_interna/Work Space Control/Grasp Planner/sfera/punti di contatto sfera/punti di contatto sfera/Subsystem2 ==
  teta/phi : Inport
  T_object : Inport  Port=2
  Constant : Constant
  Demux2 : Demux  Outputs=2
  Matrix Multiply1 : Product  Multiplication=Matrix(*)
  Mux : Mux  Inputs=2
  Mux1 : Mux  Inputs=3
  R_0EE1 : dspmtrx3/Submatrix  RowSpan=Range of rows  RowStartMode=Index  RowStartIndex=1  RowEndMode=Index  RowEndIndex=3  ColSpan=One column  ColStartMode=Index  ColStartIndex=4  ColEndMode=Index  ColEndIndex=4
  R_0EE2 : dspmtrx3/Submatrix  RowSpan=Range of rows  RowStartMode=Index  RowStartIndex=1  RowEndMode=Index  RowEndIndex=3  ColSpan=Range of columns  ColStartMode=Index  ColStartIndex=1  ColEndMode=Index  ColEndIndex=3
  Spherical to Cartesian : simulink_extras/Transformations/Spherical to Cartesian
  Sum : Sum  Inputs=|++
  Sum3 : Sum
  xyz 0 : Outport
  wires:
  [sfera_size] -> Sum/2
  [radius_spheres] -> Sum/1
  Mux/1 -> xyz 0/1
  Sum3/1 -> Mux/1
  teta/phi/1 -> Demux2/1
  Constant/1 -> Mux/2
  Demux2/2 -> Spherical to Cartesian/3
  Demux2/1 -> Spherical to Cartesian/2
  Sum/1 -> Spherical to Cartesian/1
  Spherical to Cartesian/1 -> Mux1/1
  Spherical to Cartesian/2 -> Mux1/2
  Spherical to Cartesian/3 -> Mux1/3
  Mux1/1 -> Matrix Multiply1/2
  R_0EE2/1 -> Matrix Multiply1/1
  T_object/1 -> R_0EE2/1, R_0EE1/1
  R_0EE1/1 -> Sum3/2
  Matrix Multiply1/1 -> Sum3/1

== system Simulatore_COMAU_UBHAND_IV_manipolazione_interna/Work Space Control/Grasp Planner/sfera/punti di contatto sfera/punti di contatto sfera/Subsystem3 ==
  teta/phi : Inport
  T_object : Inport  Port=2
  Constant : Constant
  Demux2 : Demux  Outputs=2
  Matrix Multiply1 : Product  Multiplication=Matrix(*)
  Mux : Mux  Inputs=2
  Mux1 : Mux  Inputs=3
  R_0EE1 : dspmtrx3/Submatrix  RowSpan=Range of rows  RowStartMode=Index  RowStartIndex=1  RowEndMode=Index  RowEndIndex=3  ColSpan=One column  ColStartMode=Index  ColStartIndex=4  ColEndMode=Index  ColEndIndex=4
  R_0EE2 : dspmtrx3/Submatrix  RowSpan=Range of rows  RowStartMode=Index  RowStartIndex=1  RowEndMode=Index  RowEndIndex=3  ColSpan=Range of columns  ColStartMode=Index  ColStartIndex=1  ColEndMode=Index  ColEndIndex=3
  Spherical to Cartesian : simulink_extras/Transformations/Spherical to Cartesian
  Sum : Sum  Inputs=|++
  Sum3 : Sum
  xyz 0 : Outport
  wires:
  Matrix Multiply1/1 -> Sum3/1
  R_0EE1/1 -> Sum3/2
  T_object/1 -> R_0EE1/1, R_0EE2/1
  R_0EE2/1 -> Matrix Multiply1/1
  Mux1/1 -> Matrix Multiply1/2
  Spherical to Cartesian/3 -> Mux1/3
  Spherical to Cartesian/2 -> Mux1/2
  Spherical to Cartesian/1 -> Mux1/1
  Sum/1 -> Spherical to Cartesian/1
  Demux2/1 -> Spherical to Cartesian/2
  Demux2/2 -> Spherical to Cartesian/3
  Constant/1 -> Mux/2
  teta/phi/1 -> Demux2/1
  Sum3/1 -> Mux/1
  Mux/1 -> xyz 0/1
  [radius_spheres] -> Sum/1
  [sfera_size] -> Sum/2

== system Simulatore_COMAU_UBHAND_IV_manipolazione_interna/Work Space Control/Grasp Planner/sfera/punti di contatto sfera/punti di contatto sfera/Subsystem4 ==
  teta/phi : Inport
  T_object : Inport  Port=2
  Constant : Constant
  Demux2 : Demux  Outputs=2
  Matrix Multiply1 : Product  Multiplication=Matrix(*)
  Mux : Mux  Inputs=2
  Mux1 : Mux  Inputs=3
  R_0EE1 : dspmtrx3/Submatrix  RowSpan=Range of rows  RowStartMode=Index  RowStartIndex=1  RowEndMode=Index  RowEndIndex=3  ColSpan=One column  ColStartMode=Index  ColStartIndex=4  ColEndMode=Index  ColEndIndex=4
  R_0EE2 : dspmtrx3/Submatrix  RowSpan=Range of rows  RowStartMode=Index  RowStartIndex=1  RowEndMode=Index  RowEndIndex=3  ColSpan=Range of columns  ColStartMode=Index  ColStartIndex=1  ColEndMode=Index  ColEndIndex=3
  Spherical to Cartesian : simulink_extras/Transformations/Spherical to Cartesian
  Sum : Sum  Inputs=|++
  Sum3 : Sum
  xyz 0 : Outport
  wires:
  [sfera_size] -> Sum/2
  [radius_spheres] -> Sum/1
  Mux/1 -> xyz 0/1
  Sum3/1 -> Mux/1
  teta/phi/1 -> Demux2/1
  Constant/1 -> Mux/2
  Demux2/2 -> Spherical to Cartesian/3
  Demux2/1 -> Spherical to Cartesian/2
  Sum/1 -> Spherical to Cartesian/1
  Spherical to Cartesian/1 -> Mux1/1
  Spherical to Cartesian/2 -> Mux1/2
  Spherical to Cartesian/3 -> Mux1/3
  Mux1/1 -> Matrix Multiply1/2
  R_0EE2/1 -> Matrix Multiply1/1
  T_object/1 -> R_0EE2/1, R_0EE1/1
  R_0EE1/1 -> Sum3/2
  Matrix Multiply1/1 -> Sum3/1

== system Simulatore_COMAU_UBHAND_IV_manipolazione_interna/Work Space Control/Inverse_kinematic/Inverse_kinematic_comau/R_0index/T_0index/Direct_kinematic_index ==
  Joints_reference : Inport
  R_0f : Inport  Port=2
  Constant : Constant  Value=[alpha_f(2,1) alpha_f(2,2) alpha_f(2,3) alpha_f(2,4)]
  Constant2 : Constant  Value=[a_f(2,1) a_f(2,2) a_f(2,3) a_f(2,4)]
  Degrees to Radians : simulink_extras/Transformations/Degrees to Radians
  Demux : Demux
  Demux1 : Demux
  Demux2 : Demux
  Demux3 : Demux  Outputs=3
  Display3 : Display
  Gain : Gain  Gain=0.7
  Matrix Multiply{,1..3} (4 alike) : Product  Multiplication=Matrix(*)
  R_0EE{,1..3} (4 alike) : dspmtrx3/Submatrix  RowSpan=Range of rows  RowStartMode=Index  RowStartIndex=1  RowEndMode=Index  RowEndIndex=3  ColSpan=Range of columns  ColStartMode=Index  ColStartIndex=1  ColEndMode=Index  ColEndIndex=3
  Subsystem : Subsystem
  Subsystem1 : Subsystem
  Subsystem2 : Subsystem
  Subsystem3 : Subsystem
  Vector Concatenate : Concatenate  Mode=Vector
  R_0index : Outport
  wires:
  Degrees to Radians/1 -> Demux/1
  Vector Concatenate/1 -> Display3/1, Degrees to Radians/1
  Gain/1 -> Vector Concatenate/2
  Demux3/3 -> Gain/1
  Joints_reference/1 -> Vector Concatenate/1, Demux3/1
  Demux1/4 -> Subsystem3/2(alpha)
  Demux1/3 -> Subsystem2/2(alpha)
  Demux1/2 -> Subsystem1/2(alpha)
  Demux1/1 -> Subsystem/2(alpha)
  Demux2/4 -> Subsystem3/3(a)
  Demux2/3 -> Subsystem2/3(a)
  Demux2/2 -> Subsystem1/3(a)
  Demux2/1 -> Subsystem/3(a)
  Demux/4 -> Subsystem3/1(q)
  Demux/3 -> Subsystem2/1(q)
  Demux/2 -> Subsystem1/1(q)
  Demux/1 -> Subsystem/1(q)
  Constant2/1 -> Demux2/1
  Matrix Multiply2/1 -> R_0index/1
  Matrix Multiply1/1 -> Matrix Multiply2/1
  Subsystem3/1(Out1) -> R_0EE3/1
  Subsystem2/1(Out1) -> R_0EE2/1
  Matrix Multiply/1 -> Matrix Multiply1/1
  Subsystem1/1(Out1) -> R_0EE1/1
  Subsystem/1(Out1) -> R_0EE/1
  Constant/1 -> Demux1/1
  R_0f/1 -> Matrix Multiply3/1
  Matrix Multiply3/1 -> Matrix Multiply/1
  R_0EE/1 -> Matrix Multiply3/2
  R_0EE1/1 -> Matrix Multiply/2
  R_0EE2/1 -> Matrix Multiply1/2
  R_0EE3/1 -> Matrix Multiply2/2

== system Simulatore_COMAU_UBHAND_IV_manipolazione_interna/Work Space Control/Inverse_kinematic/Inverse_kinematic_comau/R_0index/T_0index/Direct_kinematic_wrist ==
  R_0H : Inport
  wrist_angles[1..2] : Inport  Port=2
  Demux3 : Demux  Outputs=2
  Mux : Mux  Inputs=2
  T_6_8 : Subsystem
  R_08 : Outport
  wires:
  T_6_8/1(R_08) -> R_08/1
  wrist_angles[1..2]/1 -> Demux3/1
  Mux/1 -> T_6_8/1(wrist_angles[1..2])
  Demux3/2 -> Mux/2
  Demux3/1 -> Mux/1
  R_0H/1 -> T_6_8/2(R_0H)

== system Simulatore_COMAU_UBHAND_IV_manipolazione_interna/Work Space Control/Inverse_kinematic/Inverse_kinematic_comau/R_0index/T_6_index_^-1/Direct_kinematic_index1 ==
  Joints_reference : Inport
  T_0f : Inport  Port=2
  Constant : Constant  Value=[alpha_f(2,1) alpha_f(2,2) alpha_f(2,3) alpha_f(2,4)]
  Constant2 : Constant  Value=[a_f(2,1) a_f(2,2) a_f(2,3) a_f(2,4)]
  Degrees to Radians : simulink_extras/Transformations/Degrees to Radians
  Demux : Demux
  Demux1 : Demux
  Demux2 : Demux
  Demux3 : Demux  Outputs=3
  Display3 : Display
  Gain : Gain  Gain=0.7
  Matrix Multiply{,1..3} (4 alike) : Product  Multiplication=Matrix(*)
  Subsystem : Subsystem
  Subsystem1 : Subsystem
  Subsystem2 : Subsystem
  Subsystem3 : Subsystem
  Vector Concatenate : Concatenate  Mode=Vector
  T_6_index : Outport
  wires:
  Degrees to Radians/1 -> Demux/1
  Vector Concatenate/1 -> Display3/1, Degrees to Radians/1
  Gain/1 -> Vector Concatenate/2
  Demux3/3 -> Gain/1
  Joints_reference/1 -> Vector Concatenate/1, Demux3/1
  Demux1/4 -> Subsystem3/2(alpha)
  Demux1/3 -> Subsystem2/2(alpha)
  Demux1/2 -> Subsystem1/2(alpha)
  Demux1/1 -> Subsystem/2(alpha)
  Demux2/4 -> Subsystem3/3(a)
  Demux2/3 -> Subsystem2/3(a)
  Demux2/2 -> Subsystem1/3(a)
  Demux2/1 -> Subsystem/3(a)
  Demux/4 -> Subsystem3/1(q)
  Demux/3 -> Subsystem2/1(q)
  Demux/2 -> Subsystem1/1(q)
  Demux/1 -> Subsystem/1(q)
  Constant2/1 -> Demux2/1
  Matrix Multiply2/1 -> T_6_index/1
  Matrix Multiply1/1 -> Matrix Multiply2/1
  Subsystem3/1(Out1) -> Matrix Multiply2/2
  Subsystem2/1(Out1) -> Matrix Multiply1/2
  Matrix Multiply/1 -> Matrix Multiply1/1
  Subsystem1/1(Out1) -> Matrix Multiply/2
  Subsystem/1(Out1) -> Matrix Multiply3/2
  Constant/1 -> Demux1/1
  T_0f/1 -> Matrix Multiply3/1
  Matrix Multiply3/1 -> Matrix Multiply/1

== system Simulatore_COMAU_UBHAND_IV_manipolazione_interna/Work Space Control/Inverse_kinematic/Inverse_kinematic_comau/R_0index/T_6_index_^-1/Direct_kinematic_wrist1 ==
  T_6H : Inport
  wrist_angles[1..2] : Inport  Port=2
  Demux3 : Demux  Outputs=2
  Mux : Mux  Inputs=2
  T_6_8 : Subsystem
  T_68 : Outport
  wires:
  T_6_8/1(T_68) -> T_68/1
  wrist_angles[1..2]/1 -> Demux3/1
  Mux/1 -> T_6_8/1(wrist_angles[1..2])
  Demux3/2 -> Mux/2
  Demux3/1 -> Mux/1
  T_6H/1 -> T_6_8/2(T_6H)

== system Simulatore_COMAU_UBHAND_IV_manipolazione_interna/Work Space Control/Inverse_kinematic/Inverse_kinematic_comau/R_0index/T_6_index_^-1/T^-1 ==
  T : Inport
  -R'*v : Gain  Gain=-1
  Constant1 : Constant  Value=[0 0 0 1]  VectorParams1D=off
  Matrix Concatenation : Concatenate  Mode=Multidimensional array  ConcatenateDimension=2
  Matrix Concatenation1 : Concatenate  Mode=Multidimensional array
  R : dspmtrx3/Submatrix  RowSpan=Range of rows  RowStartMode=Index  RowStartIndex=1  RowEndMode=Index  RowEndIndex=3  ColSpan=Range of columns  ColStartMode=Index  ColStartIndex=1  ColEndMode=Index  ColEndIndex=3
  R' : PermuteDimensions
  R'*v : Product  Multiplication=Matrix(*)
  v : dspmtrx3/Submatrix  RowSpan=Range of rows  RowStartMode=Index  RowStartIndex=1  RowEndMode=Index  RowEndIndex=3  ColSpan=One column  ColStartMode=Index  ColStartIndex=4  ColEndMode=Index  ColEndIndex=3
  T^-1 : Outport
  wires:
  T/1 -> R/1, v/1
  R/1 -> R'/1
  R'/1 -> R'*v/1, Matrix Concatenation/1
  v/1 -> R'*v/2
  R'*v/1 -> -R'*v/1
  Constant1/1 -> Matrix Concatenation1/2
  Matrix Concatenation/1 -> Matrix Concatenation1/1
  -R'*v/1 -> Matrix Concatenation/2
  Matrix Concatenation1/1 -> T^-1/1

== system Simulatore_COMAU_UBHAND_IV_manipolazione_interna/Work Space Control/Inverse_kinematic/Inverse_kinematic_comau/R_0index/T_6_object^-1/Direct_kinematic_index1 ==
  Joints_reference : Inport
  T_0f : Inport  Port=2
  Constant : Constant  Value=[alpha_f(2,1) alpha_f(2,2) alpha_f(2,3) alpha_f(2,4)]
  Constant2 : Constant  Value=[a_f(2,1) a_f(2,2) a_f(2,3) a_f(2,4)]
  Degrees to Radians : simulink_extras/Transformations/Degrees to Radians
  Demux : Demux
  Demux1 : Demux
  Demux2 : Demux
  Demux3 : Demux  Outputs=3
  Display3 : Display
  Gain : Gain  Gain=0.7
  Matrix Multiply{,1..3} (4 alike) : Product  Multiplication=Matrix(*)
  Subsystem : Subsystem
  Subsystem1 : Subsystem
  Subsystem2 : Subsystem
  Subsystem3 : Subsystem
  Vector Concatenate : Concatenate  Mode=Vector
  T_6_index : Outport
  wires:
  Matrix Multiply3/1 -> Matrix Multiply/1
  T_0f/1 -> Matrix Multiply3/1
  Constant/1 -> Demux1/1
  Subsystem/1(Out1) -> Matrix Multiply3/2
  Subsystem1/1(Out1) -> Matrix Multiply/2
  Matrix Multiply/1 -> Matrix Multiply1/1
  Subsystem2/1(Out1) -> Matrix Multiply1/2
  Subsystem3/1(Out1) -> Matrix Multiply2/2
  Matrix Multiply1/1 -> Matrix Multiply2/1
  Matrix Multiply2/1 -> T_6_index/1
  Constant2/1 -> Demux2/1
  Demux/1 -> Subsystem/1(q)
  Demux/2 -> Subsystem1/1(q)
  Demux/3 -> Subsystem2/1(q)
  Demux/4 -> Subsystem3/1(q)
  Demux2/1 -> Subsystem/3(a)
  Demux2/2 -> Subsystem1/3(a)
  Demux2/3 -> Subsystem2/3(a)
  Demux2/4 -> Subsystem3/3(a)
  Demux1/1 -> Subsystem/2(alpha)
  Demux1/2 -> Subsystem1/2(alpha)
  Demux1/3 -> Subsystem2/2(alpha)
  Demux1/4 -> Subsystem3/2(alpha)
  Joints_reference/1 -> Demux3/1, Vector Concatenate/1
  Demux3/3 -> Gain/1
  Gain/1 -> Vector Concatenate/2
  Vector Concatenate/1 -> Degrees to Radians/1, Display3/1
  Degrees to Radians/1 -> Demux/1

== system Simulatore_COMAU_UBHAND_IV_manipolazione_interna/Work Space Control/Inverse_kinematic/Inverse_kinematic_comau/R_0index/T_6_object^-1/Direct_kinematic_wrist1 ==
  T_6H : Inport
  wrist_angles[1..2] : Inport  Port=2
  Demux3 : Demux  Outputs=2
  Mux : Mux  Inputs=2
  T_6_8 : Subsystem
  T_68 : Outport
  wires:
  T_6H/1 -> T_6_8/2(T_6H)
  Demux3/1 -> Mux/1
  Demux3/2 -> Mux/2
  Mux/1 -> T_6_8/1(wrist_angles[1..2])
  wrist_angles[1..2]/1 -> Demux3/1
  T_6_8/1(T_68) -> T_68/1

== system Simulatore_COMAU_UBHAND_IV_manipolazione_interna/Work Space Control/Inverse_kinematic/Inverse_kinematic_comau/R_0index/T_6_object^-1/T^-1 ==
  T : Inport
  -R'*v : Gain  Gain=-1
  Constant1 : Constant  Value=[0 0 0 1]  VectorParams1D=off
  Matrix Concatenation : Concatenate  Mode=Multidimensional array  ConcatenateDimension=2
  Matrix Concatenation1 : Concatenate  Mode=Multidimensional array
  R : dspmtrx3/Submatrix  RowSpan=Range of rows  RowStartMode=Index  RowStartIndex=1  RowEndMode=Index  RowEndIndex=3  ColSpan=Range of columns  ColStartMode=Index  ColStartIndex=1  ColEndMode=Index  ColEndIndex=3
  R' : PermuteDimensions
  R'*v : Product  Multiplication=Matrix(*)
  v : dspmtrx3/Submatrix  RowSpan=Range of rows  RowStartMode=Index  RowStartIndex=1  RowEndMode=Index  RowEndIndex=3  ColSpan=One column  ColStartMode=Index  ColStartIndex=4  ColEndMode=Index  ColEndIndex=3
  T^-1 : Outport
  wires:
  Matrix Concatenation1/1 -> T^-1/1
  -R'*v/1 -> Matrix Concatenation/2
  Matrix Concatenation/1 -> Matrix Concatenation1/1
  Constant1/1 -> Matrix Concatenation1/2
  R'*v/1 -> -R'*v/1
  v/1 -> R'*v/2
  R'/1 -> Matrix Concatenation/1, R'*v/1
  R/1 -> R'/1
  T/1 -> v/1, R/1

== system Simulatore_COMAU_UBHAND_IV_manipolazione_interna/Work Space Control/Inverse_kinematic/Inverse_kinematic_comau/R_0index/T_index_object/T^-1 ==
  T : Inport
  -R'*v : Gain  Gain=-1
  Constant1 : Constant  Value=[0 0 0 1]  VectorParams1D=off
  Matrix Concatenation : Concatenate  Mode=Multidimensional array  ConcatenateDimension=2
  Matrix Concatenation1 : Concatenate  Mode=Multidimensional array
  R : dspmtrx3/Submatrix  RowSpan=Range of rows  RowStartMode=Index  RowStartIndex=1  RowEndMode=Index  RowEndIndex=3  ColSpan=Range of columns  ColStartMode=Index  ColStartIndex=1  ColEndMode=Index  ColEndIndex=3
  R' : PermuteDimensions
  R'*v : Product  Multiplication=Matrix(*)
  v : dspmtrx3/Submatrix  RowSpan=Range of rows  RowStartMode=Index  RowStartIndex=1  RowEndMode=Index  RowEndIndex=3  ColSpan=One column  ColStartMode=Index  ColStartIndex=4  ColEndMode=Index  ColEndIndex=3
  T^-1 : Outport
  wires:
  T/1 -> R/1, v/1
  R/1 -> R'/1
  R'/1 -> R'*v/1, Matrix Concatenation/1
  v/1 -> R'*v/2
  R'*v/1 -> -R'*v/1
  Constant1/1 -> Matrix Concatenation1/2
  Matrix Concatenation/1 -> Matrix Concatenation1/1
  -R'*v/1 -> Matrix Concatenation/2
  Matrix Concatenation1/1 -> T^-1/1

== system Simulatore_COMAU_UBHAND_IV_manipolazione_interna/Work Space Control/Inverse_kinematic/Inverse_kinematic_comau/Subsystem/q5 appartiene a [-180 0]/q4 ==
  R_36 : Inport
  Gain : Gain  Gain=-1
  Gain1 : Gain  Gain=-1
  P06(1) : dspmtrx3/Submatrix  RowSpan=One row  RowStartMode=Index  RowStartIndex=2  RowEndMode=Index  RowEndIndex=3  ColSpan=One column  ColStartMode=Index  ColStartIndex=3  ColEndMode=Offset from last  ColEndIndex=1
  P06(1)1 : dspmtrx3/Submatrix  RowSpan=One row  RowStartMode=Index  RowStartIndex=1  RowEndMode=Index  RowEndIndex=3  ColSpan=One column  ColStartMode=Index  ColStartIndex=3  ColEndMode=Offset from last  ColEndIndex=1
  Trigonometric Function : Trigonometry  Operator=atan2
  q_4 : Outport
  wires:
  Trigonometric Function/1 -> q_4/1
  P06(1)1/1 -> Gain1/1
  P06(1)/1 -> Gain/1
  R_36/1 -> P06(1)1/1, P06(1)/1
  Gain/1 -> Trigonometric Function/1
  Gain1/1 -> Trigonometric Function/2

== system Simulatore_COMAU_UBHAND_IV_manipolazione_interna/Work Space Control/Inverse_kinematic/Inverse_kinematic_comau/Subsystem/q5 appartiene a [-180 0]/q5 ==
  R_36 : Inport
  Add : Sum
  Gain : Gain  Gain=-1
  Math Function : Math  Operator=sqrt  SaturateOnIntegerOverflow=on
  Math Function1 : Math  Operator=square  SaturateOnIntegerOverflow=on
  Math Function2 : Math  Operator=square  SaturateOnIntegerOverflow=on
  P06(1) : dspmtrx3/Submatrix  RowSpan=One row  RowStartMode=Index  RowStartIndex=1  RowEndMode=Index  RowEndIndex=3  ColSpan=One column  ColStartMode=Index  ColStartIndex=3  ColEndMode=Offset from last  ColEndIndex=1
  P06(1)1 : dspmtrx3/Submatrix  RowSpan=One row  RowStartMode=Index  RowStartIndex=2  RowEndMode=Index  RowEndIndex=3  ColSpan=One column  ColStartMode=Index  ColStartIndex=3  ColEndMode=Offset from last  ColEndIndex=1
  P06(1)2 : dspmtrx3/Submatrix  RowSpan=One row  RowStartMode=Index  RowStartIndex=3  RowEndMode=Index  RowEndIndex=3  ColSpan=One column  ColStartMode=Index  ColStartIndex=3  ColEndMode=Offset from last  ColEndIndex=1
  Trigonometric Function : Trigonometry  Operator=atan2
  q_5 : Outport
  wires:
  Trigonometric Function/1 -> q_5/1
  Math Function/1 -> Gain/1
  P06(1)2/1 -> Trigonometric Function/2
  Add/1 -> Math Function/1
  Math Function2/1 -> Add/2
  Math Function1/1 -> Add/1
  R_36/1 -> P06(1)2/1, P06(1)1/1, P06(1)/1
  P06(1)/1 -> Math Function1/1
  P06(1)1/1 -> Math Function2/1
  Gain/1 -> Trigonometric Function/1
